simulink model "dds_cwg_32k_nb_ch_inject_sim"  (R2018a) (continued)
== system dds_cwg_32k_nb_ch_inject_sim == (continued)
      | disp(['Z^-', num2str(csp_latency)]);
  we_delay126 : Subsystem  Tag=cmac_delinked  mask(pipeline){csp_latency=2}
      mask prompts: csp_latency "Latency"
      mask help:
      | An explicitly laid-out delay line to avoid mapping to SRL16s
      | for use in pipelining to help achieve timing closure.
      mask init:
      | pipeline_init(gcb,'latency',csp_latency);
      settings: AttributesFormatString=Latency = %<latency>
      mask Display:
      | port_label('input', 1, 'd');
      | port_label('output', 1, 'q');
      | disp(['Z^-', num2str(csp_latency)]);
  we_delay13 : Subsystem  Tag=cmac_delinked  mask(pipeline){csp_latency=2}
      mask prompts: csp_latency "Latency"
      mask help:
      | An explicitly laid-out delay line to avoid mapping to SRL16s
      | for use in pipelining to help achieve timing closure.
      mask init:
      | pipeline_init(gcb,'latency',csp_latency);
      settings: AttributesFormatString=Latency = %<latency>
      mask Display:
      | port_label('input', 1, 'd');
      | port_label('output', 1, 'q');
      | disp(['Z^-', num2str(csp_latency)]);
  we_delay37 : Subsystem  Tag=cmac_delinked  mask(pipeline){csp_latency=2}
      mask prompts: csp_latency "Latency"
      mask help:
      | An explicitly laid-out delay line to avoid mapping to SRL16s
      | for use in pipelining to help achieve timing closure.
      mask init:
      | pipeline_init(gcb,'latency',csp_latency);
      settings: AttributesFormatString=Latency = %<latency>
      mask Display:
      | port_label('input', 1, 'd');
      | port_label('output', 1, 'q');
      | disp(['Z^-', num2str(csp_latency)]);
  we_delay38 : Subsystem  Tag=cmac_delinked  mask(pipeline){csp_latency=2}
      mask prompts: csp_latency "Latency"
      mask help:
      | An explicitly laid-out delay line to avoid mapping to SRL16s
      | for use in pipelining to help achieve timing closure.
      mask init:
      | pipeline_init(gcb,'latency',csp_latency);
      settings: AttributesFormatString=Latency = %<latency>
      mask Display:
      | port_label('input', 1, 'd');
      | port_label('output', 1, 'q');
      | disp(['Z^-', num2str(csp_latency)]);
  we_delay39 : Subsystem  Tag=cmac_delinked  mask(pipeline){csp_latency=2}
      mask prompts: csp_latency "Latency"
      mask help:
      | An explicitly laid-out delay line to avoid mapping to SRL16s
      | for use in pipelining to help achieve timing closure.
      mask init:
      | pipeline_init(gcb,'latency',csp_latency);
      settings: AttributesFormatString=Latency = %<latency>
      mask Display:
      | port_label('input', 1, 'd');
      | port_label('output', 1, 'q');
      | disp(['Z^-', num2str(csp_latency)]);
  we_delay41 : Subsystem  Tag=cmac_delinked  mask(pipeline){csp_latency=2}
      mask prompts: csp_latency "Latency"
      mask help:
      | An explicitly laid-out delay line to avoid mapping to SRL16s
      | for use in pipelining to help achieve timing closure.
      mask init:
      | pipeline_init(gcb,'latency',csp_latency);
      settings: AttributesFormatString=Latency = %<latency>
      mask Display:
      | port_label('input', 1, 'd');
      | port_label('output', 1, 'q');
      | disp(['Z^-', num2str(csp_latency)]);
  we_delay47 : Subsystem  Tag=cmac_delinked  mask(pipeline){csp_latency=2}
      mask prompts: csp_latency "Latency"
      mask help:
      | An explicitly laid-out delay line to avoid mapping to SRL16s
      | for use in pipelining to help achieve timing closure.
      mask init:
      | pipeline_init(gcb,'latency',csp_latency);
      settings: AttributesFormatString=Latency = %<latency>
      mask Display:
      | port_label('input', 1, 'd');
      | port_label('output', 1, 'q');
      | disp(['Z^-', num2str(csp_latency)]);
  we_delay48 : Subsystem  Tag=cmac_delinked  mask(pipeline){csp_latency=2}
      mask prompts: csp_latency "Latency"
      mask help:
      | An explicitly laid-out delay line to avoid mapping to SRL16s
      | for use in pipelining to help achieve timing closure.
      mask init:
      | pipeline_init(gcb,'latency',csp_latency);
      settings: AttributesFormatString=Latency = %<latency>
      mask Display:
      | port_label('input', 1, 'd');
      | port_label('output', 1, 'q');
      | disp(['Z^-', num2str(csp_latency)]);
  we_delay49 : Subsystem  Tag=cmac_delinked  mask(pipeline){csp_latency=2}
      mask prompts: csp_latency "Latency"
      mask help:
      | An explicitly laid-out delay line to avoid mapping to SRL16s
      | for use in pipelining to help achieve timing closure.
      mask init:
      | pipeline_init(gcb,'latency',csp_latency);
      settings: AttributesFormatString=Latency = %<latency>
      mask Display:
      | port_label('input', 1, 'd');
      | port_label('output', 1, 'q');
      | disp(['Z^-', num2str(csp_latency)]);
  we_delay50 : Subsystem  Tag=cmac_delinked  mask(pipeline){csp_latency=2}
      mask prompts: csp_latency "Latency"
      mask help:
      | An explicitly laid-out delay line to avoid mapping to SRL16s
      | for use in pipelining to help achieve timing closure.
      mask init:
      | pipeline_init(gcb,'latency',csp_latency);
      settings: AttributesFormatString=Latency = %<latency>
      mask Display:
      | port_label('input', 1, 'd');
      | port_label('output', 1, 'q');
      | disp(['Z^-', num2str(csp_latency)]);
  wng0 : Subsystem  mask{seed1=hex2dec('12345678'), seed2=hex2dec('87654321'), seed3=hex2dec('45612378'), seed4=hex2dec('85274163')}
      mask prompts: seed1 "Seed 1"; seed2 "Seed 2"; seed3 "Seed 3"; seed4 "Seed 4"
  xeng_match : Subsystem  Tag=xps:sw_reg  AncestorBlock=xps_library/Memory/software_register  mask(swreg){io_dir=From Processor, io_delay=0, init_val=0, sample_period=1, names=[val], bitwidths=[3], bin_pts=0, arith_types=0, sim_port=on, show_format=on}
      mask prompts: io_dir "I/O direction"; io_delay "I/O delay"; init_val "Initial Value"; sample_period "Sample period"; names "Bitfield names [msb...lsb]"; bitwidths "Bitfield widths"; bin_pts "Bitfield binary pts"; arith_types "Bitfield types, ufix=0, fix=1, bool=2"; sim_port "Provide sim input/output?"; show_format "Print format string?"
      mask help: A 32-bit software-accessible register. Can be divided into bitfields of varying widths and types using the fields in the block mask.
      mask init:
      | swreg_init(gcb)
      settings: AttributesFormatString=1input:uf3.0 = 3 bits
      mask TypeOptions io_dir: To Processor | From Processor
      mask Callback io_dir:
      | mask_enables = get_param(gcb, 'MaskEnables');
      | mask_names = get_param(gcb, 'MaskNames');
      | if strcmp(get_param(gcb, 'io_dir'), 'To Processor'),
      |     mask_enables{ismember(mask_names, 'sample_period')} = 'off';
      | else
      |     mask_enables{ismember(mask_names, 'sample_period')} = 'on';
      | end
      | set_param(gcb, 'MaskEnables', mask_enables);
      mask DialogControl ParameterTabVar0 Prompt: Setup
  xsg_core_config : xps_library/Platforms/SKARAB  Tag=xps:xsg  hw_sys=SKARAB:xc7vx690t  clk_src=sys_clk  clk_rate=238  sample_period=1  synthesis_tool=XST
      settings: SourceProductName=CASPER XPS Blockset
  tag scope: cnt_rst=global, ct_board_hmc_offset=global, ct_chans_per_board=global, ct_chans_per_x=global, ct_chans_per_x_recip=global, ct_fake_data_error=global, ct_freq_gen_offset=global, ct_num_x_boards=global, ct_num_x_boards_recip=global, ct_num_xeng=global, ct_num_xeng_recip=global, ct_rst_err_latch=global, ct_tag_insert_sel=global, ct_tvg_en2=global, ct_tvg_en=global, ct_x_per_board=global, ct_x_per_board_recip=global, fft_fwd_inv=global, force_gbe_txen=global, local_time_capture=global, local_time_src=global, obuf_read_gap=global, pfb_bypass=global, snap_reord_mux_we_sel=global, snap_stop_mux_sel=global, sys_rst=global, time80_48=global, ts_tvg_en=global, tvgtone_level0=global, tvgtone_level1=global, tvgtone_p0=global, tvgtone_p1=global
  wires:
  Gateway Out20/1 -> To Workspace20/1
  DDC/1(ddc_sync) -> [ddc_sync]
  DDC/3(ddc_pol1) -> [ddc_pol1]
  DDC/4(ddc_dv) -> [ddc_dvalid]
  Gateway Out65/1 -> To Workspace69/1
  [ddc_dvalid] -> Gateway Out65/1
  [ddc_sync] -> Gateway Out21/1
  Gateway Out21/1 -> To Workspace23/1
  DDC/2(ddc_pol0) -> [ddc_pol0]
  Counter10/1 -> Relational5/1, Relational2/1
  Logical17/1 -> Gateway Out37/1, Register/2, Counter10/1
  [sys_rst] -> Logical17/1
  Relational5/1 -> Logical17/2
  Gateway Out19/1 -> To Workspace11/1
  fft_fwd_inv/1(in_fwd_inv) -> pipeline234/1(d)
  pipeline234/1(q) -> [fft_fwd_inv]
  pipeline223/1(q) -> bus_expand5/1(bus_in)
  From15/1 -> pipeline2/1(d), pipeline216/1(d)
  pipeline216/1(q) -> snap_dds/5(in_dv)
  From16/1 -> pipeline13/1(d), pipeline217/1(d)
  pipeline217/1(q) -> snap_dds/6(in_sync)
  pipeline13/1(q) -> snap_dds/8(trig)
  pipeline11/1(q) -> bus_expand2/1(bus_in)
  From18/1 -> pipeline1/1(d), pipeline9/1(d)
  From21/1 -> pipeline11/1(d)
  bus_expand2/4(out5) -> snap_cwg/4(in_p0_d3)
  bus_expand2/5(out4) -> snap_cwg/5(in_p0_d4)
  bus_expand2/1(msb_out8) -> snap_cwg/1(in_p0_d0)
  bus_expand2/2(out7) -> snap_cwg/2(in_p0_d1)
  bus_expand2/3(out6) -> snap_cwg/3(in_p0_d2)
  bus_expand2/6(out3) -> snap_cwg/6(in_p0_d5)
  bus_expand2/7(out2) -> snap_cwg/7(in_p0_d6)
  bus_expand2/8(lsb_out1) -> snap_cwg/8(in_p0_d7)
  pipeline9/1(q) -> snap_cwg/9(in_dv)
  From20/1 -> pipeline12/1(d), pipeline10/1(d)
  pipeline10/1(q) -> snap_cwg/10(in_sync)
  pipeline12/1(q) -> snap_cwg/12(trig)
  Constant4/1 -> gap_offset/1(sim_1)
  Constant55/1 -> AddSub3/2
  AddSub3/1 -> Gateway Out39/1, Relational5/2
  gap_offset/1(in_offset) -> pipeline14/1(d)
  Constant21/1 -> gbe0/8(rx_overrun_ack)
  Constant20/1 -> gbe0/7(rx_ack)
  From17/1 -> pipeline99/1(d)
  From27/1 -> pipeline31/1(d)
  pipeline31/1(q) -> gbe0/9(debug_rst)
  gbe0/13(rx_dest_ip) -> pipeline115/1(d)
  gbe0/14(rx_dest_port) -> pipeline116/1(d)
  From24/1 -> pipeline/1(d)
  pipeline/1(q) -> rx_dest_ip/1(out_reg)
  gbe0/1(led_up) -> pipeline100/1(d)
  From25/1 -> pipeline20/1(d)
  gbe0/2(led_rx) -> pipeline101/1(d)
  From46/1 -> pipeline22/1(d)
  gbe0/3(led_tx) -> pipeline102/1(d)
  From47/1 -> pipeline51/1(d)
  gbe0/4(tx_afull) -> pipeline103/1(d)
  gbe0/5(tx_overflow) -> pipeline104/1(d)
  gbe0/12(rx_overrun) -> pipeline114/1(d)
  pipeline20/1(q) -> gbe_status/1(out_led_up)
  pipeline22/1(q) -> gbe_status/2(out_led_rx)
  pipeline51/1(q) -> gbe_status/3(out_led_tx)
  gbe0/8(rx_source_ip) -> pipeline110/1(d)
  gbe0/9(rx_source_port) -> pipeline111/1(d)
  gbe0/6(rx_data) -> pipeline108/1(d)
  gbe0/10(rx_end_of_frame) -> pipeline112/1(d)
  gbe0/11(rx_bad_frame) -> pipeline113/1(d)
  From98/1 -> pipeline98/1(d)
  From94/1 -> pipeline97/1(d)
  From29/1 -> pipeline96/1(d)
  Logical3/1 -> gbe0/6(tx_end_of_frame)
  From97/1 -> Logical2/2
  From96/1 -> Logical3/2
  Delay4/1 -> Logical2/1, Logical3/1
  From99/1 -> Delay4/2
  pipeline96/1(q) -> gbe0/5(tx_dest_port)
  pipeline97/1(q) -> gbe0/4(tx_dest_ip)
  pipeline98/1(q) -> gbe0/2(tx_data)
  pipeline99/1(q) -> gbe0/1(rst)
  Logical5/1 -> Delay4/1
  pipeline100/1(q) -> Goto7/1
  pipeline101/1(q) -> Goto9/1
  pipeline102/1(q) -> Goto19/1
  pipeline103/1(q) -> Goto20/1
  pipeline104/1(q) -> Goto21/1
  pipeline108/1(q) -> Goto25/1
  pipeline110/1(q) -> Goto23/1
  pipeline111/1(q) -> Goto24/1
  pipeline112/1(q) -> Goto31/1
  pipeline113/1(q) -> Goto33/1
  pipeline114/1(q) -> Goto22/1
  pipeline115/1(q) -> Goto10/1
  pipeline116/1(q) -> Goto13/1
  Logical2/1 -> gbe0/3(tx_valid)
  gbe0/7(rx_valid) -> pipeline62/1(d)
  pipeline62/1(q) -> Goto27/1
  From153/1 -> Logical5/2
  From145/1 -> Logical7/2
  Logical7/1 -> Logical5/1
  edge_detect7/1(out) -> pipeline24/1(d)
  control/7(in_cnt_rst) -> edge_detect5/1(in)
  edge_detect5/1(out) -> pipeline18/1(d)
  control/3(in_gbe_rst) -> pipeline25/1(d)
  control/1(in_sys_rst) -> pipeline23/1(d)
  pipeline23/1(q) -> [sys_rst]
  pipeline24/1(q) -> [clr_status]
  pipeline25/1(q) -> [gbe_rst]
  pipeline26/1(q) -> [gbe_txen]
  pipeline17/1(q) -> [tvg_adc0_en]
  pipeline18/1(q) -> [cnt_rst]
  control/5(in_tvg_adc0) -> pipeline17/1(d)
  edge_detect6/1(out) -> pipeline38/1(d)
  pipeline38/1(q) -> [gbe_cnt_rst]
  control/8(in_gbe_cnt_rst) -> edge_detect6/1(in)
  control/4(in_gbe_txen) -> pipeline26/1(d)
  control/2(in_status_clr) -> edge_detect7/1(in)
  pipeline43/1(q) -> [adc_snap_arm]
  control/9(in_adc_snap_arm) -> pipeline43/1(d)
  control/10(in_adc_snap_trig_select) -> pipeline39/1(d)
  pipeline39/1(q) -> [adc_snap_trig_source]
  pipeline59/1(q) -> [ct_tvg_en]
  control/11(in_ct_tvg_en) -> pipeline59/1(d)
  pipeline52/1(q) -> [up_dbg_rst]
  control/12(in_unpack_debug_reset) -> pipeline52/1(d)
  control/13(in_cd_bypass) -> pipeline131/1(d)
  pipeline131/1(q) -> [cd_bypass]
  pipeline93/1(q) -> [tvg_adc1_en]
  control/6(in_tvg_adc1) -> pipeline93/1(d)
  control/14(in_gbe_stopmux) -> pipeline27/1(d)
  pipeline27/1(q) -> [gbe_stopmux]
  control/15(in_pfb_bypass) -> pipeline66/1(d)
  pipeline66/1(q) -> [pfb_bypass]
  control/16(in_ts_tvg_en) -> pipeline148/1(d)
  pipeline148/1(q) -> [ts_tvg_en]
  control/17(in_force_gbe_txen) -> pipeline21/1(d)
  pipeline21/1(q) -> [force_gbe_txen]
  control/18(in_snap_stop_mux_sel) -> pipeline127/1(d)
  control/19(in_snap_reord_mux_we_sel) -> pipeline128/1(d)
  pipeline127/1(q) -> [snap_stop_mux_sel]
  pipeline128/1(q) -> [snap_reord_mux_we_sel]
  control/20(in_local_time_source) -> pipeline16/1(d)
  edge_detect8/1(out) -> pipeline164/1(d)
  pipeline164/1(q) -> [local_time_capture]
  pipeline16/1(q) -> [local_time_src]
  control/21(in_local_time_capture) -> edge_detect8/1(in)
  control/22(in_time_diff_check_en) -> pipeline47/1(d)
  pipeline47/1(q) -> [time_diff_check_en]
  control/23(in_auto_rst_enable) -> pipeline132/1(d)
  pipeline132/1(q) -> [auto_rst_enable]
  pipeline155/1(q) -> [pipeline_stall]
  control/24(in_pipeline_stall) -> pipeline155/1(d)
  From28/1 -> Inverter/1
  Inverter/1 -> Logical7/1
  Constant18/1 -> Goto28/1
  Constant22/1 -> Goto26/1
  Constant23/1 -> Goto18/1
  Constant24/1 -> Goto/1
  Constant25/1 -> Goto16/1
  Constant26/1 -> Goto17/1
  Constant27/1 -> fft_fwd_inv/1(sim_1)
  fft_shift/1(in_fft_shift) -> fft_shift_munge/1(din)
  pipeline15/1(q) -> [fft_shift]
  Constant28/1 -> fft_shift/1(sim_1)
  pipeline1/1(q) -> snap_cwg/11(we)
  pipeline2/1(q) -> snap_dds/7(we)
  Constant65/1 -> ts_ss_lock/1(sim_1)
  ts_ss_lock/1(in_en) -> pipeline180/1(d)
  pipeline180/1(q) -> Goto174/1
  arm_ts_ss_lock/1(in_en) -> pipeline181/1(d)
  pipeline181/1(q) -> edge_detect12/1(in)
  Constant69/1 -> arm_ts_ss_lock/1(sim_1)
  edge_detect12/1(out) -> Goto175/1
  Constant70/1 -> ss_trig_time_in_lsw/1(sim_1)
  ss_trig_time_in_lsw/1(in_time) -> pipeline183/1(d)
  arm_ss_ts_rst/1(in_rst) -> pipeline184/1(d)
  pipeline184/1(q) -> edge_detect15/1(in)
  Constant68/1 -> arm_ss_ts_rst/1(sim_1)
  edge_detect15/1(out) -> Goto178/1
  ss_trig_time_in_msw/1(in_time) -> pipeline3/1(d)
  Constant71/1 -> ss_trig_time_in_msw/1(sim_1)
  pipeline3/1(q) -> Concat/1
  pipeline183/1(q) -> Concat/2
  Concat/1 -> Goto179/1
  Counter/1 -> [time80_48]
  From11/1 -> impulse gen/1(dds_in)
  From36/1 -> impulse gen/2(ddc_dv_in)
  From35/1 -> impulse gen/3(ddc_sync_in)
  Constant1/1 -> impulse_en/1(sim_1)
  impulse_en/1(in_en) -> impulse gen/5(imp_en)
  From2/1 -> impulse gen/4(cwg_in)
  [ddc_sync_in] -> DDC/1(sync)
  [ddc_dv_in] -> DDC/2(dvalid)
  [osc_cmplx] -> DDC/6(osc1), DDC/4(osc0)
  [osc_temp_dv] -> Delay3/1
  cwg_cos/2(data_out) -> Delay8/1
  [osc_freq] -> dds_freq_idx_offset_calc/1(ddc_freq)
  dds_freq_idx_offset_calc/1(dds_fftbin_offset) -> [dds_fft_phase_offset]
  [osc_freq] -> cwg_cos/2(freq)
  freq_cwg_osc/1(in_frequency) -> [osc_freq]
  Gateway Out10/1 -> To Workspace12/1
  Gateway Out1/1 -> To Workspace10/1
  bus_expand3/1(msb_out8) -> Gateway Out10/1
  bus_expand3/2(out7) -> Gateway Out1/1
  bus_expand3/3(out6) -> Gateway Out24/1
  bus_expand3/4(out5) -> Gateway Out25/1
  bus_expand3/5(out4) -> Gateway Out26/1
  bus_expand3/6(out3) -> Gateway Out27/1
  bus_expand3/7(out2) -> Gateway Out28/1
  bus_expand3/8(lsb_out1) -> Gateway Out29/1
  [osc_cos_ddc0] -> re_im_interleave/1(In1)
  [osc_sin_ddc0] -> re_im_interleave/2(In2)
  re_im_interleave/1(Out1) -> [osc_cmplx]
  From1/1 -> bus_expand3/1(bus_in)
  Gateway Out24/1 -> To Workspace24/1
  Gateway Out25/1 -> To Workspace25/1
  Gateway Out26/1 -> To Workspace26/1
  Gateway Out27/1 -> To Workspace27/1
  Gateway Out28/1 -> To Workspace28/1
  Gateway Out29/1 -> To Workspace29/1
  bus_expand4/1(msb_out2) -> Gateway Out22/1
  Gateway Out22/1 -> To Workspace22/1
  bus_expand4/2(lsb_out1) -> Gateway Out23/1
  Gateway Out23/1 -> To Workspace1/1
  [ddc_pol0] -> bus_expand4/1(bus_in)
  [ddc_pol0_in] -> DDC/5(pol1_data), DDC/3(pol0_data)
  [cwg_awgn] -> complexify/1(In1)
  complexify/1(Out1) -> [cwg_cmplx]
  freq_cwg_input/1(in_frequency) -> Gateway Out34/1, cwg2/2(freq)
  [sys_rst] -> cwg2/4(rst)
  [sys_rst] -> Register2/2
  edge_detect2/1(out) -> Gateway Out19/1, cwg2/1(sync)
  Delay2/1 -> Gateway Out20/1, cwg2/3(dv)
  Gateway Out30/1 -> To Workspace30/1
  Gateway Out31/1 -> To Workspace31/1
  Delay3/1 -> cwg_cos/3(dv)
  [sys_rst] -> cwg_cos/4(rst)
  Constant3/1 -> cwg_cos/1(sync)
  cwg_sin/2(data_out) -> Delay9/1
  [sys_rst] -> cwg_sin/4(rst)
  Delay5/1 -> cwg_sin/3(dv)
  [osc_temp_dv] -> Delay5/1
  [osc_freq] -> cwg_sin/2(freq)
  Constant5/1 -> cwg_sin/1(sync)
  Gateway Out32/1 -> To Workspace32/1
  [ddc_of] -> Gateway Out32/1
  DDC/5(ddc_of) -> [ddc_of]
  fft_shift_munge/1(dout) -> pipeline15/1(d)
  cwg_scale/1(in_frequency) -> Gateway Out33/1, cwg2/5(scale)
  Constant7/1 -> cwg_cos/5(scale)
  Constant15/1 -> cwg_sin/5(scale)
  Constant16/1 -> cwg_scale/1(sim_1)
  Gateway Out33/1 -> To Workspace33/1
  Gateway Out3/1 -> To Workspace4/1
  bus_expand18/1(msb_out8) -> Gateway Out3/1
  Gateway Out2/1 -> To Workspace2/1
  bus_expand18/2(out7) -> Gateway Out2/1
  Gateway Out4/1 -> To Workspace3/1
  bus_expand18/3(out6) -> Gateway Out4/1
  Gateway Out5/1 -> To Workspace5/1
  bus_expand18/4(out5) -> Gateway Out5/1
  Gateway Out6/1 -> To Workspace6/1
  bus_expand18/5(out4) -> Gateway Out6/1
  Gateway Out7/1 -> To Workspace7/1
  bus_expand18/6(out3) -> Gateway Out7/1
  Gateway Out8/1 -> To Workspace8/1
  bus_expand18/7(out2) -> Gateway Out8/1
  Gateway Out9/1 -> To Workspace9/1
  bus_expand18/8(lsb_out1) -> Gateway Out9/1
  From/1 -> bus_expand18/1(bus_in)
  Gateway Out12/1 -> To Workspace13/1
  Gateway Out11/1 -> To Workspace14/1
  Gateway Out13/1 -> To Workspace15/1
  Gateway Out14/1 -> To Workspace16/1
  Gateway Out15/1 -> To Workspace17/1
  Gateway Out16/1 -> To Workspace18/1
  Gateway Out17/1 -> To Workspace19/1
  Gateway Out18/1 -> To Workspace21/1
  bus_expand1/1(msb_out8) -> Gateway Out12/1
  bus_expand1/2(out7) -> Gateway Out11/1
  bus_expand1/3(out6) -> Gateway Out13/1
  bus_expand1/4(out5) -> Gateway Out14/1
  bus_expand1/5(out4) -> Gateway Out15/1
  bus_expand1/6(out3) -> Gateway Out16/1
  bus_expand1/7(out2) -> Gateway Out17/1
  bus_expand1/8(lsb_out1) -> Gateway Out18/1
  From12/1 -> bus_expand1/1(bus_in)
  Register2/1 -> Delay1/1
  From41/1 -> pipeline223/1(d)
  From42/1 -> pipeline6/1(d), pipeline5/1(d)
  pipeline6/1(q) -> snap_dds1/5(in_dv)
  From43/1 -> pipeline7/1(d), pipeline4/1(d)
  pipeline7/1(q) -> snap_dds1/6(in_sync)
  pipeline4/1(q) -> snap_dds1/8(trig)
  pipeline5/1(q) -> snap_dds1/7(we)
  bus_expand5/1(msb_out8) -> snap_dds/1(in_p0_d0)
  bus_expand5/2(out7) -> snap_dds/2(in_p0_d1)
  bus_expand5/3(out6) -> snap_dds/3(in_p0_d2)
  bus_expand5/4(out5) -> snap_dds/4(in_p0_d3)
  bus_expand5/5(out4) -> snap_dds1/1(in_p0_d0)
  bus_expand5/6(out3) -> snap_dds1/2(in_p0_d1)
  bus_expand5/7(out2) -> snap_dds1/3(in_p0_d2)
  bus_expand5/8(lsb_out1) -> snap_dds1/4(in_p0_d3)
  Gateway Out34/1 -> To Workspace34/1
  Constant17/1 -> White Gaussian Noise/1
  Constant19/1 -> White Gaussian Noise/2
  White Gaussian Noise/1 -> Reinterpret/1
  Reinterpret/1 -> Slice/1
  pipeline14/1(q) -> Mux/2, AddSub2/1
  Constant30/1 -> gap_sel/1(sim_1)
  gap_sel/1(in_sel) -> pipeline8/1(d)
  pipeline8/1(q) -> Mux/1
  Mux/1 -> Register/1
  Register/1 -> AddSub3/1, Gateway Out38/1
  Gateway Out37/1 -> To Workspace37/1
  Gateway Out38/1 -> To Workspace38/1
  Gateway Out39/1 -> To Workspace39/1
  Reinterpret1/1 -> Slice1/1
  White Gaussian Noise/2 -> Reinterpret1/1
  [pkt_len] -> Relational2/2
  Constant32/1 -> pkt_len_sel/1(sim_1)
  pkt_len_sel/1(in_sel) -> pipeline19/1(d)
  pipeline19/1(q) -> Mux1/1
  Constant33/1 -> pkt_len/1(sim_1)
  pipeline28/1(q) -> AddSub1/1, Mux1/2
  Gateway Out40/1 -> To Workspace40/1
  Mux1/1 -> Gateway Out40/1, [pkt_len]
  AddSub1/1 -> Mux1/3
  Slice1/1 -> AddSub1/2
  Delay1/1 -> edge_detect2/1(in)
  pkt_len/1(in_len) -> pipeline28/1(d)
  Slice/1 -> AddSub2/2
  AddSub2/1 -> Mux/3
  cwg2/3(dv_out) -> Delay6/1
  cwg2/2(data_out) -> Delay7/1
  Delay6/1 -> [cwg_dv], Gateway Out31/1
  Delay7/1 -> [cwg80]
  Delay8/1 -> [osc_cos_ddc0]
  Delay9/1 -> [osc_sin_ddc0]
  wb_prog_delay/3(dvalid) -> Goto39/1
  From197/1 -> pipeline33/1(d)
  tl_cd_bs0/4(dout) -> Goto75/1
  wb_prog_delay/2(data_out) -> Goto37/1
  Constant37/1 -> tl_cd_bs0/7(misci)
  From155/1 -> wb_prog_delay/2(delay_val)
  From49/1 -> pipeline88/1(d)
  From100/1 -> wb_prog_delay/4(en)
  From50/1 -> wb_prog_delay/3(din)
  From196/1 -> pipeline32/1(d)
  From163/1 -> pipeline89/1(d)
  pipeline30/1(q) -> tl_cd_bs0/8(arm)
  pipeline88/1(q) -> tl_cd_bs0/5(din)
  tl_cd_bs0/6(time) -> Goto38/1
  pipeline29/1(q) -> tl_cd_bs0/2(current_time)
  From167/1 -> wb_prog_delay/5(load_val)
  From160/1 -> pipeline29/1(d)
  From166/1 -> wb_prog_delay/1(sync)
  tl_cd_bs0/1(value) -> Goto77/1
  tl_cd_bs0/5(dvalid) -> Goto76/1
  tl_cd_bs0/3(sync_out) -> Goto78/1
  pipeline32/1(q) -> tl_cd_bs0/9(load_immediate)
  From162/1 -> pipeline84/1(d)
  tl_cd_bs0/2(enable) -> Goto79/1
  tl_cd_bs0/7(misco) -> Terminator12/1
  pipeline78/1(q) -> tl_cd_bs0/3(load_value)
  pipeline89/1(q) -> tl_cd_bs0/6(en)
  From156/1 -> pipeline77/1(d)
  pipeline84/1(q) -> tl_cd_bs0/4(sync_in)
  wb_prog_delay/1(sync_out) -> Goto49/1
  pipeline77/1(q) -> tl_cd_bs0/1(rst)
  From161/1 -> pipeline78/1(d)
  From195/1 -> pipeline30/1(d)
  pipeline33/1(q) -> tl_cd_bs0/10(load_time)
  Constant/1 -> Goto44/1
  From44/1 -> Goto40/1
  From45/1 -> Goto42/1
  From48/1 -> Goto43/1
  bus_expand6/8(lsb_out1) -> Gateway Out48/1
  Gateway Out48/1 -> To Workspace49/1
  bus_expand6/7(out2) -> Gateway Out47/1
  Gateway Out47/1 -> To Workspace48/1
  bus_expand6/6(out3) -> Gateway Out46/1
  Gateway Out46/1 -> To Workspace47/1
  bus_expand6/5(out4) -> Gateway Out45/1
  Gateway Out45/1 -> To Workspace46/1
  bus_expand6/4(out5) -> Gateway Out44/1
  Gateway Out44/1 -> To Workspace45/1
  bus_expand6/3(out6) -> Gateway Out43/1
  Gateway Out43/1 -> To Workspace44/1
  bus_expand6/2(out7) -> Gateway Out41/1
  Gateway Out41/1 -> To Workspace42/1
  bus_expand6/1(msb_out8) -> Gateway Out42/1
  Gateway Out42/1 -> To Workspace43/1
  From51/1 -> bus_expand6/1(bus_in)
  Gateway Out49/1 -> To Workspace41/1
  From53/1 -> Gateway Out49/1
  Gateway Out50/1 -> To Workspace50/1
  From52/1 -> Gateway Out50/1
  Delay10/1 -> Register2/1, Register2/3, Delay2/1
  tl_cd1/6(time) -> Goto47/1
  tl_cd1/1(value) -> Goto64/1
  Constant45/1 -> Concat15/2
  tl_cd0/2(enable) -> Goto62/1
  Delay23/1 -> cd_hmc/7(din1)
  From184/1 -> pipeline41/1(d)
  From57/1 -> Delay28/1
  From70/1 -> Delay23/1
  From81/1 -> pipeline35/1(d), pipeline75/1(d)
  cd_hmc/5(hmc_error) -> Goto51/1
  From121/1 -> Delay21/1
  cd_hmc/1(sync_out) -> Delay11/1, sync_mangle/1(sync_in)
  Delay29/1 -> cd_hmc/2(din)
  From104/1 -> Delay27/1
  From188/1 -> pipeline91/1(d)
  From86/1 -> pipeline40/1(d), pipeline76/1(d)
  From_pfb_d5/1 -> Concat15/1
  From54/1 -> pipeline37/1(d)
  sync_mangle/2(dout0) -> Delay111/1
  From55/1 -> pipeline36/1(d)
  cd_hmc/3(dout1) -> sync_mangle/3(din1)
  Delay25/1 -> cd_hmc/5(load_val)
  pipeline45/1(q) -> tl_cd1/10(load_time)
  Delay21/1 -> cd_hmc/9(delay_val1)
  From90/1 -> pipeline73/1(d)
  tl_cd1/7(misco) -> Terminator2/1
  Concat15/1 -> pipeline34/1(d), Gateway Out36/1, pipeline74/1(d)
  tl_cd0/5(dvalid) -> Goto58/1
  tl_cd0/7(misco) -> Terminator/1
  pipeline40/1(q) -> tl_cd1/6(en)
  sync_mangle/1(sync_out) -> Goto100/1
  tl_cd0/6(time) -> Goto46/1
  pipeline73/1(q) -> tl_cd0/3(load_value)
  pipeline35/1(q) -> tl_cd1/1(rst)
  sync_mangle/3(dout1) -> Delay109/1
  pipeline36/1(q) -> tl_cd1/3(load_value)
  pipeline41/1(q) -> tl_cd1/8(arm)
  From123/1 -> Delay24/1
  pipeline76/1(q) -> tl_cd0/6(en)
  From193/1 -> pipeline42/1(d), pipeline72/1(d)
  Delay111/1 -> Goto95/1
  Delay30/1 -> cd_hmc/1(sync_in)
  tl_cd1/5(dvalid) -> Goto68/1
  pipeline34/1(q) -> tl_cd1/2(current_time)
  pipeline44/1(q) -> tl_cd1/9(load_immediate)
  cd_hmc/4(dvalid) -> sync_mangle/4(en)
  From109/1 -> Delay29/1
  pipeline37/1(q) -> tl_cd1/5(din)
  cd_hmc/2(dout0) -> sync_mangle/2(din)
  pipeline91/1(q) -> tl_cd0/9(load_immediate)
  tl_cd1/4(dout) -> Goto67/1
  pipeline75/1(q) -> tl_cd0/1(rst)
  Delay11/1 -> Goto45/1
  pipeline71/1(q) -> tl_cd0/5(din)
  Gateway Out36/1 -> To Workspace36/1
  Constant44/1 -> tl_cd1/7(misci)
  From190/1 -> pipeline92/1(d)
  From56/1 -> pipeline44/1(d)
  tl_cd1/3(sync_out) -> Goto66/1
  Delay22/1 -> cd_hmc/8(en1)
  From146/1 -> Delay22/1
  From73/1 -> pipeline45/1(d)
  From111/1 -> Delay25/1
  Delay27/1 -> cd_hmc/3(en)
  From108/1 -> Delay30/1
  pipeline92/1(q) -> tl_cd0/10(load_time)
  pipeline42/1(q) -> tl_cd1/4(sync_in)
  tl_cd0/4(dout) -> Goto60/1
  pipeline74/1(q) -> tl_cd0/2(current_time)
  tl_cd0/1(value) -> Goto63/1
  From187/1 -> pipeline90/1(d)
  pipeline90/1(q) -> tl_cd0/8(arm)
  pipeline72/1(q) -> tl_cd0/4(sync_in)
  Delay28/1 -> cd_hmc/10(load_val1)
  Constant39/1 -> tl_cd0/7(misci)
  Delay26/1 -> cd_hmc/4(delay_val)
  From85/1 -> pipeline71/1(d)
  tl_cd1/2(enable) -> Goto65/1
  Delay109/1 -> Goto92/1
  tl_cd0/3(sync_out) -> Goto57/1
  From112/1 -> Delay26/1
  Delay108/1 -> Goto96/1
  Delay24/1 -> cd_hmc/6(sync_in1)
  sync_mangle/4(dvalid) -> Delay108/1
  From58/1 -> Goto50/1, Goto48/1
  [ddc_sync_in] -> [sync80]
  [ddc_dv_in] -> [valid80]
  Constant46/1 -> [time80]
  Concat12/1 -> pipeline170/1(d)
  Concat14/1 -> [ld_val1]
  Delay119/1 -> Concat12/1
  delta_delay_whole1/1(in_initial) -> Reinterpret10/1
  delta_delay_frac0/1(in_initial) -> Reinterpret21/1
  Constant36/1 -> phase0/1(sim_1)
  delay_frac1/1(in_initial) -> Reinterpret5/1
  Reinterpret10/1 -> Delay123/1
  Concat9/1 -> pipeline174/1(d)
  Constant60/1 -> phase1/1(sim_1)
  Shift2/1 -> Concat12/2
  Shift3/1 -> Concat3/2
  Constant47/1 -> delay_whole1/1(sim_1)
  pipeline175/1(q) -> Concat14/3
  Delay121/1 -> pipeline172/1(d)
  delta_delay_frac1/1(in_initial) -> Reinterpret11/1
  Constant63/1 -> delta_delay_whole1/1(sim_1)
  Reinterpret2/1 -> Delay120/1
  Constant75/1 -> phase0/2(sim_2)
  Delay125/1 -> pipeline176/1(d)
  Constant77/1 -> delta_delay_whole0/1(sim_1)
  Reinterpret12/1 -> Delay125/1
  pipeline176/1(q) -> Concat14/4
  Concat11/1 -> pipeline169/1(d)
  pipeline174/1(q) -> Concat14/2
  Reinterpret18/1 -> Shift1/1
  Reinterpret20/1 -> Delay119/1
  Delay124/1 -> pipeline175/1(d)
  Shift1/1 -> Concat11/2
  Reinterpret21/1 -> Shift2/1
  Constant76/1 -> delay_frac0/1(sim_1)
  Concat3/1 -> pipeline173/1(d)
  Concat22/1 -> [ld_val0]
  phase0/2(in_delta) -> Reinterpret3/1
  pipeline170/1(q) -> Concat22/2
  Constant61/1 -> phase1/2(sim_2)
  Delay120/1 -> pipeline171/1(d)
  Delay118/1 -> Concat11/1
  pipeline171/1(q) -> Concat22/3
  delay_whole0/1(in_initial) -> Reinterpret19/1
  pipeline169/1(q) -> Concat22/1
  pipeline172/1(q) -> Concat22/4
  delay_whole1/1(in_initial) -> Reinterpret6/1
  Reinterpret9/1 -> Delay124/1
  Constant78/1 -> delta_delay_frac0/1(sim_1)
  delta_delay_whole0/1(in_initial) -> Reinterpret20/1
  Constant64/1 -> delta_delay_frac1/1(sim_1)
  Delay122/1 -> Concat3/1
  pipeline173/1(q) -> Concat14/1
  Reinterpret19/1 -> Delay118/1
  Shift4/1 -> Concat9/2
  delay_frac0/1(in_initial) -> Reinterpret18/1
  Delay123/1 -> Concat9/1
  phase1/1(in_initial) -> Reinterpret9/1
  Reinterpret11/1 -> Shift4/1
  Reinterpret3/1 -> Delay121/1
  phase0/1(in_initial) -> Reinterpret2/1
  Constant62/1 -> delay_frac1/1(sim_1)
  Reinterpret5/1 -> Shift3/1
  Reinterpret6/1 -> Delay122/1
  phase1/2(in_delta) -> Reinterpret12/1
  Constant35/1 -> delay_whole0/1(sim_1)
  Concat6/1 -> pipeline136/1(d)
  tl_cd1_control0/2(in_load_immediate) -> pipeline138/1(d)
  Constant49/1 -> tl_cd1_control0/2(sim_2)
  Delay13/1 -> tl_cd1_control0/1(sim_1)
  Constant40/1 -> tl_cd0_control0/3(sim_3)
  Constant51/1 -> Delay13/1
  tl_cd1_control0/3(in_load_time_msw) -> Concat8/1
  Constant48/1 -> tl_cd1_control0/3(sim_3)
  pipeline143/1(q) -> [tl_cd1_load_time]
  pipeline138/1(q) -> [tl_cd1_load_immediate]
  Delay12/1 -> tl_cd0_control0/1(sim_1)
  Constant42/1 -> tl_cd0_control/1(sim_1)
  Concat8/1 -> pipeline143/1(d)
  tl_cd1_control0/1(in_arm) -> pipeline137/1(d)
  pipeline137/1(q) -> [tl_cd1_arm]
  tl_cd0_control0/1(in_arm) -> pipeline134/1(d)
  tl_cd0_control/1(in_load_time_lsw) -> Concat6/2
  pipeline136/1(q) -> [tl_cd0_load_time]
  Constant50/1 -> tl_cd1_control/1(sim_1)
  pipeline134/1(q) -> [tl_cd0_arm]
  tl_cd0_control0/2(in_load_immediate) -> pipeline135/1(d)
  tl_cd0_control0/3(in_load_time_msw) -> Concat6/1
  pipeline135/1(q) -> [tl_cd0_load_immediate]
  Constant43/1 -> Delay12/1
  Constant41/1 -> tl_cd0_control0/2(sim_2)
  tl_cd1_control/1(in_load_time_lsw) -> Concat8/2
  [cwg_cmplx] -> [ddc_pol0_in]
  [ddc_sync] -> nb_pfb/1(sync)
  [ddc_pol0] -> nb_pfb/2(pol0)
  [ddc_pol1] -> nb_pfb/3(pol1)
  [ddc_dvalid] -> nb_pfb/4(dvalid)
  [fft_shift] -> nb_pfb/5(fft_shift)
  [osc_temp_dv] -> Delay10/1
  Constant38/1 -> [osc_temp_dv]
  Constant34/1 -> freq_cwg_osc/1(sim_1)
  Constant58/1 -> freq_cwg_input/1(sim_1)
  [pfb_pol0] -> Gateway Out95/1, bus_expand7/1(bus_in)
  Gateway Out53/1 -> To Workspace51/1
  bus_expand7/2(lsb_out1) -> Gateway Out53/1
  Gateway Out52/1 -> To Workspace52/1
  bus_expand7/1(msb_out2) -> Gateway Out52/1
  Gateway Out51/1 -> To Workspace53/1
  [pfb_sync] -> Gateway Out51/1
  [pfb_dv] -> Gateway Out54/1
  Gateway Out54/1 -> To Workspace54/1
  wng0/1(bus_out) -> [wng]
  pipeline46/1(q) -> [wng_scale0]
  [wng_scale0] -> wng0/1(scale_16_14)
  man_rst_wng0/1(in_rst) -> Logical20/2
  Logical20/1 -> pipeline198/1(d)
  pipeline198/1(q) -> wng0/2(rst)
  scale_wng0/1(in_scale) -> Gateway Out55/1, pipeline46/1(d)
  Constant72/1 -> man_rst_wng0/1(sim_1)
  Constant73/1 -> scale_wng0/1(sim_1)
  Gateway Out55/1 -> To Workspace55/1
  bus_addsub/1(dout) -> bus_convert2/1(din)
  bus_convert2/1(dout) -> [cwg_awgn]
  [cwg80] -> bus_addsub/1(a)
  [wng] -> bus_addsub/2(b)
  bus_addsub/2(misco) -> bus_convert2/2(misci)
  cwg2/1(sync_out) -> Gateway Out30/1, [cwg_sync]
  Concat1/1 -> bus_addsub/3(misci)
  [cwg_sync] -> Concat1/1
  [cwg_dv] -> Concat1/2
  bus_expand8/1(msb_out2) -> [ddc_sync_in]
  bus_expand8/2(lsb_out1) -> [ddc_dv_in]
  bus_convert2/2(misco) -> bus_expand8/1(bus_in)
  [sys_rst] -> Logical20/1
  Delay127/1 -> Delay144/1
  Delay130/1 -> Delay142/1
  nb_pfb/1(sync_out) -> Delay127/1
  Delay143/1 -> Delay146/1
  nb_pfb/2(dv_out) -> Delay128/1
  nb_pfb/3(pol0_out) -> Delay130/1
  Delay145/1 -> [pfb_sync]
  Delay144/1 -> Delay145/1
  nb_pfb/6(pfb_ch_out) -> Delay39/1
  Delay128/1 -> Delay143/1
  Delay146/1 -> [pfb_dv]
  Delay39/1 -> Convert7/1
  Gateway Out95/1 -> To Workspace95/1
  Delay142/1 -> Delay38/1
  Delay38/1 -> [pfb_pol0]
  Convert7/1 -> Delay40/1
  Delay40/1 -> [pfb_ch_out]
  Gateway Out35/1 -> To Workspace35/1
  [pfb_ch_out] -> Gateway Out35/1
  [ld_val0] -> tl_fd0/3(load_value)
  pipeline129/1(q) -> [pfb_ch_inject]
  Gateway Out110/1 -> To Workspace110/1
  Counter53/1 -> Slice42/1, Slice43/1
  [pfb_ch_inject] -> Mux2/1
  Constant86/1 -> pfb_ch_inject/1(sim_1)
  [fdfs_d144_0] -> Delay17/1
  From513/1 -> Mux16/2
  quant1/3(dvalid) -> Terminator21/1
  calc_quant_pwr1/1(mean_pwr) -> pipeline165/1(d)
  Constant52/1 -> eq1/3(we)
  Delay140/1 -> Gateway Out67/1, Delay141/1
  [tl_delay1_load_time] -> tl_fd1/10(load_time)
  [q_d64_1] -> Delay117/1
  Delay15/1 -> demux/1(sync_in)
  fd_nb1/1(sync_out) -> Terminator24/1
  pfb_ch_inject/1(in_en) -> pipeline129/1(d)
  [fdfs_sync] -> Delay51/1, pipeline56/1(d)
  pipeline55/1(q) -> Counter4/2
  [tl_delay0_load_immediate] -> tl_fd0/9(load_immediate)
  Constant53/1 -> tl_fd0/7(misci)
  From511/1 -> Counter53/2
  Delay115/1 -> calc_quant_pwr1/2(valid_in)
  [fdfs_d144_1] -> Delay34/1
  tl_fd1/7(misco) -> Terminator20/1
  [fdfs_dv] -> Delay52/1, pipeline55/1(d)
  Delay53/1 -> quant1/1(sync_in)
  bus_expand16/1(msb_out2) -> tl_fd0/5(din), Gateway Out64/1
  [pfb_sync] -> pipeline210/1(d)
  Delay117/1 -> calc_quant_pwr1/1(adc_in)
  eq0/1(data_out) -> quant0/4(coeffs)
  Mux15/1 -> Goto192/1
  [tg_pfb_dv] -> tl_fd0/6(en)
  fd_nb0/2(dout) -> Gateway Out71/1, [fd_nb_d0], Mux2/2
  Logical34/1 -> Delay18/1
  tl_fd1/2(enable) -> fd_nb1/5(load_value)
  [pfb_pol0] -> pipeline228/1(d)
  fd_nb0/1(sync_out) -> [fd_nb_sync], Gateway Out56/1, Delay15/1
  Mux14/1 -> Goto193/1
  From510/1 -> Convert6/1
  Counter17/1 -> quant_sync_cnt/1(out_reg)
  Mux17/1 -> Goto195/1
  Slice42/1 -> Logical34/1
  pipeline165/1(q) -> quant_pwr1/1(out_reg)
  From493/1 -> Mux14/2
  Delay18/1 -> Mux14/3
  [pfb_dv] -> pipeline212/1(d)
  pipeline48/1(q) -> Counter17/2
  calc_quant_pwr0/1(mean_pwr) -> pipeline160/1(d)
  Subsystem15/1(out0) -> quant_status/1(out_pol0_or_cnt)
  From393/1 -> pipeline48/1(d)
  [pfb_pol1] -> pipeline231/1(d)
  Counter4/1 -> pipeline53/1(d)
  Constant79/1 -> eq1/2(data_in)
  pipeline212/1(q) -> time_gen_nb/5(rd_en)
  Delay50/1 -> Gateway Out70/1, quant1/3(en)
  bus_expand14/4(out5) -> Gateway Out114/1, Convert9/1
  [tl_delay0_load_time] -> tl_fd0/10(load_time)
  pipeline209/1(q) -> time_gen_nb/3(wr_val_in)
  tl_fd0/7(misco) -> Terminator3/1
  [clr_status] -> tl_fd0/1(cnt_rst), tl_fd1/1(cnt_rst)
  Delay116/1 -> calc_quant_pwr0/1(adc_in)
  quant1/4(of) -> Delay174/1
  Delay139/1 -> Gateway Out62/1, Delay140/1
  bus_expand16/2(lsb_out1) -> Gateway Out63/1, tl_fd1/5(din)
  From503/1 -> Delay19/1, Counter53/1
  pipeline57/1(q) -> Counter2/2
  Constant83/1 -> Relational3/2
  [sync80] -> pipeline207/1(d)
  Counter2/1 -> pipeline54/1(d)
  From512/1 -> Mux17/1, Mux16/1, Mux14/1, Mux15/1
  pipeline210/1(q) -> time_gen_nb/4(rd_sync_in)
  Constant84/1 -> Relational/2
  Concat2/1 -> time_gen_nb/6(rd_misc_in)
  bus_expand14/2(out7) -> Gateway Out113/1, Convert8/1
  demux/1(sync_out) -> [fdfs_sync]
  Delay16/1 -> demux/4(pol1_in)
  pipeline56/1(q) -> Counter4/1
  time_gen_nb/2(rd_dvalid) -> Gateway Out66/1, [tg_pfb_dv]
  Mux2/1 -> demux/3(pol0_in)
  [fdfs_sync] -> Delay53/1, pipeline58/1(d)
  pipeline208/1(q) -> time_gen_nb/2(wr_en)
  Gateway Out114/1 -> To Workspace114/1
  Gateway Out111/1 -> To Workspace111/1
  Relational3/1 -> Subsystem15/3(in1)
  pipeline160/1(q) -> quant_pwr0/1(out_reg)
  From419/1 -> Mux17/2
  From388/1 -> pipeline50/1(d)
  tl_fd0/1(value) -> fd_nb0/4(delay_value)
  [q_of1] -> Relational3/1
  time_gen_nb/1(rd_sync_out) -> [tg_pfb_sync]
  Constant74/1 -> eq0/3(we)
  Gateway Out57/1 -> To Workspace57/1
  Gateway Out112/1 -> To Workspace112/1
  [tl_delay1_load_immediate] -> tl_fd1/9(load_immediate)
  fd_nb1/2(dout) -> Delay16/1
  Gateway Out61/1 -> To Workspace61/1
  Delay14/1 -> demux/2(dv_in)
  quant1/1(sync_out) -> Terminator19/1
  tl_fd0/6(time) -> [fdfs_time]
  Delay34/1 -> quant1/2(din)
  pipeline231/1(q) -> Concat2/2
  Delay114/1 -> calc_quant_pwr0/2(valid_in)
  pipeline133/1(q) -> Delay139/1
  Gateway Out58/1 -> To Workspace58/1
  pipeline58/1(q) -> Counter2/1
  [tl_delay0_arm] -> tl_fd0/8(arm)
  Gateway Out56/1 -> To Workspace56/1
  Subsystem15/2(out1) -> quant_status/2(out_pol1_or_cnt)
  [tg_pfb_dv] -> tl_fd1/6(en)
  Constant54/1 -> eq0/2(data_in)
  Mux16/1 -> Goto194/1
  Gateway Out115/1 -> To Workspace115/1
  pipeline50/1(q) -> Counter17/1
  Delay19/1 -> Mux15/3
  [valid80] -> pipeline208/1(d)
  Relational/1 -> Subsystem15/2(in0)
  Mux5/1 -> [q_d64_1]
  Gateway Out59/1 -> To Workspace59/1
  pipeline53/1(q) -> eq0/1(addr)
  Convert5/1 -> Mux16/3
  [q_of0] -> Relational/1
  time_gen_nb/4(rd_misc_out) -> bus_expand16/1(bus_in)
  From509/1 -> Convert5/1
  tl_fd0/4(dout) -> Gateway Out69/1, [tlfd_d0], fd_nb0/2(din)
  [pfb_ch_inject] -> Mux5/1
  tl_fd0/3(sync_out) -> [tlfd_sync], fd_nb0/1(sync_in)
  tl_fd1/5(dvalid) -> fd_nb1/3(en)
  Gateway Out116/1 -> To Workspace116/1
  eq1/1(data_out) -> quant1/4(coeffs)
  fd_nb0/3(dvalid) -> [fd_nb_dv], Gateway Out61/1, Delay14/1
  demux/3(pol0_out) -> [fdfs_d144_0]
  tl_fd0/5(dvalid) -> [tlfd_dv], fd_nb0/3(en), Gateway Out68/1
  [osc_freq] -> [tlfd_osc], fd_nb0/6(mix_freq), fd_nb1/6(mix_freq)
  pipeline207/1(q) -> time_gen_nb/1(wr_sync_in)
  Delay17/1 -> quant0/2(din)
  fd_nb1/3(dvalid) -> Terminator25/1
  [q_dv] -> Delay115/1
  pipeline228/1(q) -> Concat2/1
  Delay52/1 -> quant0/3(en)
  quant1/2(dout) -> Mux5/2
  tl_fd1/4(dout) -> fd_nb1/2(din)
  tl_fd1/1(value) -> fd_nb1/4(delay_value)
  Delay51/1 -> quant0/1(sync_in)
  [time80_48] -> pipeline209/1(d)
  [q_dv] -> Delay114/1
  pipeline54/1(q) -> eq1/1(addr)
  demux/4(pol1_out) -> [fdfs_d144_1]
  [clr_status] -> Subsystem15/1(rst)
  [pfb_ch_out] -> pipeline133/1(d)
  tl_fd0/2(enable) -> fd_nb0/5(load_value)
  [q_d64_0] -> Delay116/1
  Gateway Out113/1 -> To Workspace113/1
  From502/1 -> Mux15/2
  Gateway Out109/1 -> To Workspace109/1
  tl_fd1/3(sync_out) -> fd_nb1/1(sync_in)
  time_gen_nb/3(rd_val_out) -> tl_fd0/2(current_time), tl_fd1/2(current_time), [pfb_time]
  Constant81/1 -> tl_fd1/7(misci)
  [tl_delay1_arm] -> tl_fd1/8(arm)
  demux/2(dv_out) -> [fdfs_dv]
  [tg_pfb_sync] -> tl_fd1/4(sync_in)
  [tg_pfb_sync] -> tl_fd0/4(sync_in)
  Convert10/1 -> Gateway Out111/1, Concat4/3
  Convert6/1 -> Mux17/3
  Convert9/1 -> Gateway Out110/1, Concat4/2
  bus_expand14/6(out3) -> Gateway Out115/1, Convert10/1
  [fdfs_d144_0] -> bus_expand14/1(bus_in)
  tl_fd1/6(time) -> Terminator17/1
  Convert8/1 -> Gateway Out109/1, Concat4/1
  quant0/3(dvalid) -> Delay171/1
  Concat4/1 -> Delay170/1
  quant0/2(dout) -> Mux4/2
  [pfb_ch_inject] -> Mux4/1
  quant0/1(sync_out) -> Delay173/1
  Delay173/1 -> Gateway Out57/1, [q_sync]
  Delay171/1 -> Gateway Out59/1, [q_dv]
  Delay170/1 -> Mux5/3, Mux4/3
  Delay172/1 -> [q_of0]
  Convert11/1 -> Gateway Out112/1, Concat4/4
  Gateway Out60/1 -> To Workspace60/1
  [ld_val1] -> tl_fd1/3(load_value)
  Delay141/1 -> Gateway Out60/1, Mux2/3
  Delay174/1 -> [q_of1]
  Mux4/1 -> Gateway Out58/1, [q_d64_0]
  Slice43/1 -> Logical34/2
  quant0/4(of) -> Delay172/1
  bus_expand14/8(lsb_out1) -> Gateway Out116/1, Convert11/1
  Delay31/1 -> Delay32/1
  nb_pfb/4(pol1_out) -> Delay20/1
  Delay20/1 -> Delay31/1
  Delay32/1 -> [pfb_pol1]
  pipeline65/1(q) -> [tl_delay1_load_immediate]
  Delay33/1 -> delay1_tl_control0/1(sim_1)
  pipeline60/1(q) -> [tl_delay0_arm]
  Concat5/1 -> pipeline63/1(d)
  delay0_tl_control0/2(in_load_immediate) -> pipeline61/1(d)
  Constant85/1 -> delay0_tl_control0/3(sim_3)
  Constant90/1 -> delay1_tl_control0/3(sim_3)
  delay1_tl_control0/1(in_arm) -> pipeline64/1(d)
  pipeline64/1(q) -> [tl_delay1_arm]
  Constant93/1 -> Delay33/1
  delay0_tl_control1/1(in_load_time_lsw) -> Concat5/2
  Constant88/1 -> delay0_tl_control1/1(sim_1)
  delay0_tl_control0/1(in_arm) -> pipeline60/1(d)
  Constant91/1 -> delay1_tl_control0/2(sim_2)
  pipeline61/1(q) -> [tl_delay0_load_immediate]
  pipeline67/1(q) -> [tl_delay1_load_time]
  Concat7/1 -> pipeline67/1(d)
  delay1_tl_control0/3(in_load_time_msw) -> Concat7/1
  delay0_tl_control0/3(in_load_time_msw) -> Concat5/1
  Constant92/1 -> delay1_tl_control1/1(sim_1)
  Constant87/1 -> delay0_tl_control0/2(sim_2)
  Constant89/1 -> Delay113/1
  delay1_tl_control0/2(in_load_immediate) -> pipeline65/1(d)
  Delay113/1 -> delay0_tl_control0/1(sim_1)
  pipeline63/1(q) -> [tl_delay0_load_time]
  delay1_tl_control1/1(in_load_time_lsw) -> Concat7/2
  Gateway Out62/1 -> To Workspace62/1
  Gateway Out63/1 -> To Workspace63/1
  Gateway Out64/1 -> To Workspace64/1
  Gateway Out66/1 -> To Workspace65/1
  Gateway Out67/1 -> To Workspace66/1
  Gateway Out68/1 -> To Workspace67/1
  Gateway Out69/1 -> To Workspace68/1
  Gateway Out71/1 -> To Workspace71/1
  Gateway Out70/1 -> To Workspace70/1
  Gateway Out72/1 -> To Workspace72/1
  [fdfs_dv] -> Delay50/1, pipeline57/1(d), Gateway Out72/1
  Slice18/1 -> [tvgtone_p0]
  tvg_tone_levels/2(in_p0i) -> bus_create2/2(in2)
  we_delay49/1(q) -> [ct_tag_insert_sel]
  [ct_pktfreq] -> Delay35/1
  Gateway Out75/1 -> To Workspace75/1
  pipeline157/1(q) -> [ct_snap_trigsel]
  we_delay117/1(q) -> [ct_freq_gen_offset]
  ct_debug/1(in_in_stopmux) -> pipeline70/1(d)
  hmc_ct/1(out_sync) -> [ct_sync]
  Delay41/1 -> bus_expand9/1(bus_in), Mux3/2
  we_delay39/1(q) -> [ct_chans_per_x]  "PER_X"
  ct_control1/2(in_num_x_recip) -> we_delay112/1(d)
  [ct_pktstart] -> Delay47/1
  time_gen1/3(rd_val_out) -> [flag_time]
  we_delay41/1(q) -> [ct_num_xeng]
  hmc_ct/4(pkt_start) -> [ct_pktstart]
  pipeline49/1(q) -> [outfreq_stopmux]
  ct_control0/5(in_tag_insert) -> we_delay49/1(d)
  Constant80/1 -> Concat13/2
  [ct_tvg_en2] -> Mux3/1
  ct_control2/1(in_chans_per_x) -> we_delay39/1(d)
  [valid80] -> Subsystem/3(dv)
  Delay59/1 -> time_gen1/6(rd_misc_in)
  ct_control0/6(in_obuf_read_gap) -> we_delay126/1(d)
  ct_control1/1(in_num_x) -> we_delay41/1(d)
  [sync80] -> time_gen1/1(wr_sync_in)
  Delay64/1 -> [flag_pktstart]
  Delay47/1 -> Gateway Out76/1, Delay54/1
  pipeline79/1(q) -> [ct_snap_wesel]
  ct_debug/5(in_out_stopmux) -> pipeline68/1(d)
  Delay/1 -> Delay48/1  "gapsync"
  Delay46/1 -> Delay49/1  "gapdata"
  [ct_dv] -> Delay37/1
  [ct_pkt_x_offset] -> Delay43/1
  [ct_pkt_x_index] -> Delay36/1
  Delay48/1 -> time_gen1/4(rd_sync_in)
  ct_debug/8(in_snap_trig) -> pipeline147/1(d)
  Delay43/1 -> Gateway Out75/1, Delay44/1
  Delay67/1 -> [flag_pktxoffset]
  [sync80] -> Subsystem/2(sync)
  Delay55/1 -> Delay61/1
  ct_control1/3(in_x_per_board) -> we_delay114/1(d)
  [time80] -> Slice2/1
  Delay37/1 -> Gateway Out91/1, tvg/2(dv), Delay46/1
  Delay65/1 -> [flag_pktfreq]
  we_delay112/1(q) -> [ct_num_xeng_recip]  "NUM_X_RECIP"
  pipeline68/1(q) -> [out_stopmux]
  Subsystem/2(ls32) -> sync_time_lsw/1(out_reg)
  we_delay48/1(q) -> [ct_fake_data_error]
  we_delay124/1(q) -> [ct_chans_per_x_recip]
  edge_detect4/1(out) -> [ct_snap_trig]
  Delay54/1 -> Delay60/1
  pipeline70/1(q) -> [in_stopmux]
  Gateway Out76/1 -> To Workspace76/1
  [q_d64_1] -> hmc_ct/4(in_d1)
  tvg_tone_targets/1(in_p0) -> Slice18/1
  Delay66/1 -> [flag_pktxindex]
  Concat13/1 -> Slice4/1, Slice11/1, [flag_epoch]
  Gateway Out73/1 -> To Workspace73/1
  Delay49/1 -> time_gen1/5(rd_en)
  pipeline122/1(q) -> [ct_snap_arm]
  Delay36/1 -> Gateway Out74/1, Delay56/1
  ct_debug/9(in_snap_wesel) -> pipeline79/1(d)
  Slice11/1 -> flag_epoch_msw/1(out_reg)
  ct_debug/10(in_snap_trigsel) -> pipeline157/1(d)
  [ct_d256] -> Delay41/1
  Delay63/1 -> Delay67/1
  Delay44/1 -> Delay63/1
  Gateway Out74/1 -> To Workspace74/1
  ct_control2/2(in_chans_per_board) -> we_delay113/1(d)
  [ct_sync] -> Delay42/1
  ct_control3/1(in_num_x_boards) -> we_delay38/1(d)
  we_delay115/1(q) -> [ct_x_per_board_recip]
  Delay62/1 -> Delay66/1
  Delay42/1 -> Gateway Out92/1, tvg/1(sync), Delay/1
  hmc_ct/8(error0) -> [ct_error]
  ct_control0/1(in_tvg_en) -> we_delay13/1(d)
  we_delay47/1(q) -> edge_detect3/1(in)
  [time80] -> Subsystem/1(time)
  Delay60/1 -> Delay64/1
  Slice2/1 -> time_gen1/3(wr_val_in)
  time_gen1/4(rd_misc_out) -> [flag_misc]
  ct_control0/7(in_chans_per_x_recip) -> we_delay124/1(d)
  we_delay50/1(q) -> [ct_tvg_en2]
  Slice36/1 -> we_delay116/1(d)
  hmc_ct/5(freq_id) -> [ct_pktfreq]
  we_delay126/1(q) -> [obuf_read_gap]
  we_delay38/1(q) -> [ct_num_x_boards]
  ct_control3/2(in_num_x_boards_recip) -> we_delay37/1(d)
  hmc_ct/7(x_pkt_index) -> [ct_pkt_x_offset]
  time_gen1/1(rd_sync_out) -> [flag_sync]
  Subsystem/1(msw) -> sync_time_msw/1(out_reg)
  Delay61/1 -> Delay65/1
  tvg_tone_levels/1(in_p0r) -> bus_create2/1(in1)
  Delay56/1 -> Delay62/1
  hmc_ct/6(x_index) -> [ct_pkt_x_index]
  time_gen1/5(epoch) -> Concat13/1
  pipeline147/1(q) -> edge_detect4/1(in)
  we_delay113/1(q) -> [ct_chans_per_board]
  [valid80] -> time_gen1/2(wr_en)
  hmc_ct/2(out_dv) -> [ct_dv]
  Slice4/1 -> flag_epoch_lsw/1(out_reg)
  pipeline69/1(q) -> [wrrd_stopmux]
  ct_debug/2(in_in_trigmux) -> pipeline150/1(d)
  pipeline150/1(q) -> [in_trigmux]
  ct_debug/4(in_obuf_stopmux) -> pipeline167/1(d)
  pipeline167/1(q) -> [obuf_stopmux]
  tvg_tone_levels/4(in_p1i) -> bus_create3/2(in2)
  ct_debug/11(in_snap_circsel) -> pipeline166/1(d)
  ct_control0/4(in_fake_error) -> we_delay48/1(d)
  ct_debug/7(in_snap_arm) -> pipeline122/1(d)
  bus_create2/1(bus_out) -> [tvgtone_level0]
  bus_create3/1(bus_out) -> [tvgtone_level1]
  tvg_tone_levels/3(in_p1r) -> bus_create3/1(in1)
  tvg_tone_targets/2(in_p1) -> Slice19/1
  edge_detect3/1(out) -> [ct_rst_err_latch]
  we_delay13/1(q) -> [ct_tvg_en]
  ct_control0/2(in_tvg_en2) -> we_delay50/1(d)
  ct_control0/3(in_rst_err_latch) -> we_delay47/1(d)
  ct_debug/3(in_wrrd_stopmux) -> pipeline69/1(d)
  ct_control1/4(in_x_per_board_recip) -> we_delay115/1(d)
  we_delay114/1(q) -> [ct_x_per_board]
  we_delay37/1(q) -> [ct_num_x_boards_recip]
  Slice19/1 -> [tvgtone_p1]
  ct_control4/1(in_ct_board_offset) -> Slice36/1
  ct_control5/1(in_ct_freq_gen_offset) -> we_delay117/1(d)
  pipeline166/1(q) -> [ct_snap_circsel]
  [q_sync] -> hmc_ct/1(in_sync)
  we_delay116/1(q) -> [ct_board_hmc_offset]
  [q_d64_0] -> hmc_ct/3(in_d0)
  ct_debug/6(in_outfreq_stopmux) -> pipeline49/1(d)
  hmc_ct/3(out_d256) -> [ct_d256]
  time_gen1/2(rd_dvalid) -> [flag_dvalid]
  Mux3/1 -> Delay59/1  "gapvalid"
  tvg1/1(data256) -> Mux3/3
  Delay35/1 -> Gateway Out73/1, tvg1/1(freq), Delay55/1
  [q_dv] -> hmc_ct/2(in_dv)
  [flag_pktfreq] -> pipeline189/1(d)
  Delay162/1 -> Delay169/1
  Gateway Out84/1 -> To Workspace84/1
  Delay154/1 -> Gateway Out77/1, snap_pack/1(in_d0)
  pipeline189/1(q) -> Delay160/1
  Gateway Out83/1 -> To Workspace83/1
  Delay57/1 -> snap_pack/9(we)
  pipeline82/1(q) -> data_change2/2(dv)
  [flag_pktxindex] -> pipeline156/1(d)
  pipeline156/1(q) -> match/2(data)
  pipeline83/1(q) -> match/3(match_value)
  Delay164/1 -> Delay147/1
  pipeline81/1(q) -> data_change2/1(data)
  Constant82/1 -> pack_dv_sel/1(sim_1)
  Gateway Out77/1 -> To Workspace77/1
  bus_expand11/1(msb_out8) -> pipeline191/1(d)
  Delay157/1 -> Delay164/1
  [flag_pktstart] -> Reinterpret7/1
  pipeline191/1(q) -> Delay162/1
  data_change2/1(trig) -> Mux6/5
  pipeline139/1(q) -> match/1(dv)
  Delay165/1 -> Delay150/1
  Delay156/1 -> Delay163/1
  [flag_misc] -> bus_expand11/1(bus_in)
  pipeline187/1(q) -> Delay158/1
  pipeline190/1(q) -> Delay161/1
  Logical1/1 -> Mux6/7
  Constant57/1 -> xeng_match/1(sim_1)
  [flag_pktxindex] -> pipeline177/1(d)
  Gateway Out79/1 -> To Workspace79/1
  data_change1/1(trig) -> Mux6/3
  Reinterpret7/1 -> pipeline81/1(d)
  Gateway Out81/1 -> To Workspace81/1
  Delay168/1 -> Delay153/1
  [flag_time] -> pipeline186/1(d)
  pack_dv_sel/1(in_sel) -> pipeline179/1(d)
  Delay167/1 -> Delay152/1
  Delay155/1 -> Mux6/4
  pipeline178/1(q) -> data_change1/2(dv)
  Gateway Out78/1 -> To Workspace78/1
  [flag_pktfreq] -> pipeline158/1(d)
  Delay147/1 -> Gateway Out84/1, snap_pack/8(in_time)
  Delay163/1 -> Delay45/1, Delay148/1
  Mux6/1 -> Delay149/1, Delay57/1
  Delay152/1 -> Gateway Out79/1, snap_pack/3(in_pktfreq)
  Delay158/1 -> Delay165/1
  [flag_pktxindex] -> pipeline188/1(d)
  Delay161/1 -> Delay168/1
  pipeline177/1(q) -> data_change1/1(data)
  [flag_pktxoffset] -> pipeline187/1(d)
  pipeline168/1(q) -> data_change/2(dv)
  Delay160/1 -> Delay167/1
  [flag_sync] -> pipeline185/1(d)
  match/1(Out1) -> Logical1/1, Mux6/6
  Delay153/1 -> Gateway Out78/1, snap_pack/2(in_pktstart)
  pipeline80/1(q) -> Delay155/1
  Delay169/1 -> Delay154/1
  pipeline186/1(q) -> Delay157/1
  [flag_dvalid] -> pipeline178/1(d), pipeline80/1(d), pipeline82/1(d), pipeline139/1(d), pipeline168/1(d)
  pipeline158/1(q) -> data_change/1(data)
  pipeline179/1(q) -> Mux6/1
  Delay159/1 -> Delay166/1
  xeng_match/1(in_val) -> pipeline83/1(d)
  Delay166/1 -> Delay151/1
  pipeline185/1(q) -> Delay156/1
  Delay148/1 -> Gateway Out83/1, snap_pack/7(in_sync)
  pipeline188/1(q) -> Delay159/1
  Gateway Out80/1 -> To Workspace80/1
  data_change/1(trig) -> Logical1/2, Mux6/2
  Gateway Out82/1 -> To Workspace82/1
  Delay149/1 -> Gateway Out82/1, snap_pack/6(in_dv)
  Delay45/1 -> snap_pack/10(trig)
  Delay150/1 -> Gateway Out81/1, snap_pack/5(in_pktoffset)
  [flag_pktstart] -> pipeline190/1(d)
  Delay151/1 -> Gateway Out80/1, snap_pack/4(in_pktindex)
  Gateway Out85/1 -> To Workspace85/1
  bus_expand9/1(msb_out16) -> Gateway Out85/1
  Gateway Out86/1 -> To Workspace86/1
  bus_expand9/2(out15) -> Gateway Out86/1
  Gateway Out87/1 -> To Workspace87/1
  bus_expand9/3(out14) -> Gateway Out87/1
  Gateway Out88/1 -> To Workspace88/1
  bus_expand9/4(out13) -> Gateway Out88/1
  Gateway Out89/1 -> To Workspace89/1
  bus_expand9/16(lsb_out1) -> Gateway Out89/1
  Gateway Out90/1 -> To Workspace90/1
  bus_expand9/15(out2) -> Gateway Out90/1
  Gateway Out91/1 -> To Workspace91/1
  Gateway Out92/1 -> To Workspace92/1
